simulink library "Renault_Library"  (Simulink 9.2)
settings: Solver=ode3  StartTime=0.0  StopTime=10.0  FixedStep=0.020  RelTol=1e-3  EnableMultiTasking=on
undefined symbols (not defined in the file or in its folder): ABC, AssystemProposal, b, chkval, CoeffA_C, CoeffB_C, CoeffMean_C, ConfTime_C, DiagDelay_C, DividePercent, FailureConfActive_C, FallingEdgeDuration_C, HighLevelThresholdVoltage_C, HighLevelVoltage_C, HighLevelVoltageTunable_C, InitValue_C, InputVoltRangeMax_C, InputVoltRangeMin_C, LowActiveContact_C, LowLevelThresholdVoltage_C, LowLevelVoltage_C, MaxOperatingVoltage_C, MinOperatingVoltage_C, mV2V_conversion, OUT1, OutOfRangeHigh_C, OutOfRangeLow_C, PinSupplySaturationMax, PinSupplySaturationMin, Res1Value_C, Res2Value_C, Res3Value_C, RisingEdgeDuration_C, RSA_CANSignalFailMonitoring, SampleTime_C, service14Table, service19Table, service22Table, service24Table, service28Table, service2ETable, service2FTable, service31Table, service87Table, SizeXStateDID2FTable, SizeXTable14, SizeXTable19, SizeXTable22, SizeXTable24, SizeXTable28, SizeXTable2E, SizeXTable2F, SizeXTable31, SizeXTable87, SizeYStateDID2FTable, SizeYTable14, SizeYTable19, SizeYTable22, SizeYTable24, SizeYTable28 ... (+22)
undefined symbols (not defined in the file): BatteryVoltage_ActivationThreshold_C, FrameStatus_AbsentValue_C, FrameStatus_ConfAbsentValue_C, MemThreshold_C, NAMEOFTHEMODEL_SAMPLE_TIME_CST, NbAbs1_C, NbConfAbs1_C, NbConfPres1_C, NbPres1_C, NOMDUSIGNAL_FaultConfTime_C, NOMDUSIGNAL_FaultRehabTime_C, NOMDUSIGNAL_switchvalue_C, NOMDUSIGNAL_unavailablevalue_C, PeriodFrame1_C, ResetTime_C, WakeUpTime_C
libraries: $bdroot x112, simulink x75
(part 30 of 32)

== stateflow chart Services ECU/Diag/RSA_DiagnosticManagement_v02.00/DIAGNOSTIC_ACTION_TOP/Diagnostic_Action ==
  state Action/
    state No_Message/
      entry: RES_DATA_NUMBER=0;
    state Response/
      entry :
      serviceAction(0);...
      DIAG_BUFFER_RESPONSE[0]=DIAG_BUFFER_REQUEST[0]+0x40;
      during :serviceAction(1);
    state Message_without_response/
      entry:
      RES_DATA_NUMBER=1;
    state Negative_Response/
      entry :
      DIAG_BUFFER_RESPONSE[0]=0x7F;...
      DIAG_BUFFER_RESPONSE[1]=DIAG_BUFFER_REQUEST[0];...
      DIAG_BUFFER_RESPONSE[2]=NEGATIVE_RES_CODE;...
      RES_DATA_NUMBER=3;
    (default) -> No_Message
    (junction 10) -> No_Message
    Negative_Response -> No_Message : [hasChanged(RES_DATA_NUMBER)]
    Response -> No_Message : [hasChanged(RES_DATA_NUMBER) ||...
        |    hasChanged(DIAG_BUFFER_REQUEST)]
    (junction 13) -> (junction 10)
    No_Message -> (junction 11) : [hasChanged(SEND_RES_MESSAGE)]
        | {
        |  DIAG_BUFFER_RESPONSE=0;...
        |
        | }
    (junction 11) -> Negative_Response [order 1] : [NEGATIVE_RES_CODE!=0]
    (junction 11) -> Response [order 3] : [NEGATIVE_RES_CODE==0 &&...
        |  SUPPRESS_POS_RES!=1]
    (junction 11) -> Message_without_response [order 2] : [NEGATIVE_RES_CODE==0 &&...
        |    SUPPRESS_POS_RES==1]
    Message_without_response -> (junction 12)
    (junction 12) -> (junction 13)
  (default) -> Action : {defineValues();}
  data: fillResponse function_input double; data_number local uint16; DIAG_BUFFER_REQUEST input uint8 size=4095; NEGATIVE_RES_CODE input uint8; SUPPRESS_POS_RES input double; DIAG_OUTPUT output Bus: busOutput size=1 =0; SEND_RES_MESSAGE input boolean; DIAG_BUFFER_RESPONSE output uint8 size=4095; RES_DATA_NUMBER output uint16; LIST_DID_SERVICE_22 input uint16 size=[2046 3]; DIAG_INPUT input Bus: busInput size=1; P2_server_max local uint16 =P2_server_max; P2server_max local uint16 =P2server_max
  function serviceAction(fillResponse):
    | function serviceAction(fillResponse)
    |     service=DIAG_BUFFER_REQUEST(1);
    |     %create a M-file for each service 
    |     switch service
    |         case (hex2dec('10'))
    |             [DIAG_OUTPUT,DIAG_BUFFER_RESPONSE,RES_DATA_NUMBER]=startDiagnosticSession(DIAG_OUTPUT,DIAG_BUFFER_RESPONSE,RES_DATA_NUMBER,DIAG_INPUT,DIAG_BUFFER_REQUEST,fillResponse);
    |             
    |         case (hex2dec('11'))
    |             [DIAG_OUTPUT,DIAG_BUFFER_RESPONSE,RES_DATA_NUMBER]= ecuReset(DIAG_OUTPUT,DIAG_BUFFER_RESPONSE,RES_DATA_NUMBER,DIAG_INPUT,DIAG_BUFFER_REQUEST,fillResponse);
    |             
    |         case (hex2dec('14'))
    |             [DIAG_OUTPUT,DIAG_BUFFER_RESPONSE,RES_DATA_NUMBER]= clearDTC(DIAG_OUTPUT,DIAG_BUFFER_RESPONSE,RES_DATA_NUMBER,DIAG_INPUT,DIAG_BUFFER_REQUEST,fillResponse);
    |             
    |         case (hex2dec('19'))
    |             subFunction = DIAG_BUFFER_REQUEST(2);
    |             [DIAG_BUFFER_RESPONSE,RES_DATA_NUMBER]=readDTCInformation(subFunction,DIAG_BUFFER_REQUEST,DIAG_BUFFER_RESPONSE,RES_DATA_NUMBER,DIAG_INPUT,fillResponse);
    |             
    |         case (hex2dec('22'))
    |             if(fillResponse==1)
    |                 data_number=uint16(0);
    |                 for current_did=1:2046
    |                     if( LIST_DID_SERVICE_22(current_did,1)~=0)
    |                         DIAG_BUFFER_RESPONSE=readDataByIdentifier(LIST_DID_SERVICE_22(current_did,1),(LIST_DID_SERVICE_22(current_did,2)-1)*8,DIAG_BUFFER_RESPONSE,DIAG_INPUT);
    |                         data_number =LIST_DID_SERVICE_22(current_did,2)+1+LIST_DID_SERVICE_22(current_did,3)+1;         
    |                     end                
    |                 end
    |                 RES_DATA_NUMBER=data_number;
    |             end
    |             
    |         case (hex2dec('23'))            
    |             [DIAG_BUFFER_RESPONSE,RES_DATA_NUMBER]= readMemoryByAddress(DIAG_BUFFER_RESPONSE,RES_DATA_NUMBER,DIAG_INPUT,DIAG_BUFFER_REQUEST,fillResponse);
    |         
    |         case (hex2dec('24'))            
    |             [DIAG_BUFFER_RESPONSE,RES_DATA_NUMBER]= readScalingDataByIdentifier(DIAG_BUFFER_RESPONSE,RES_DATA_NUMBER,DIAG_INPUT,DIAG_BUFFER_REQUEST,fillResponse);
    |               
    |         case (hex2dec('27'))
    |             [DIAG_OUTPUT,DIAG_BUFFER_RESPONSE,RES_DATA_NUMBER]= securityAccess(DIAG_OUTPUT,DIAG_BUFFER_RESPONSE,RES_DATA_NUMBER,DIAG_INPUT,DIAG_BUFFER_REQUEST,fillResponse);
    |         
    |         case (hex2dec('28'))
    |             [DIAG_OUTPUT,DIAG_BUFFER_RESPONSE,RES_DATA_NUMBER]= communicationControl(DIAG_OUTPUT,DIAG_BUFFER_RESPONSE,RES_DATA_NUMBER,DIAG_INPUT,DIAG_BUFFER_REQUEST,fillResponse);
    |         
    |         case (hex2dec('2E'))
    |             [DIAG_OUTPUT,DIAG_BUFFER_RESPONSE,RES_DATA_NUMBER]= writeDataByIdentifier(DIAG_OUTPUT,DIAG_BUFFER_RESPONSE,RES_DATA_NUMBER,DIAG_INPUT,DIAG_BUFFER_REQUEST,fillResponse);
    |         
    |         case (hex2dec('2F'))
    |             [DIAG_OUTPUT,DIAG_BUFFER_RESPONSE,RES_DATA_NUMBER]= inputOutputControl(DIAG_OUTPUT,DIAG_BUFFER_RESPONSE,RES_DATA_NUMBER,DIAG_INPUT,DIAG_BUFFER_REQUEST,fillResponse);
    |         
    |         case (hex2dec('31'))
    |             [DIAG_OUTPUT,DIAG_BUFFER_RESPONSE,RES_DATA_NUMBER]= startRoutine(DIAG_OUTPUT,DIAG_BUFFER_RESPONSE,RES_DATA_NUMBER,DIAG_INPUT,DIAG_BUFFER_REQUEST,fillResponse);
    |         
    |         case (hex2dec('3E'))
    |             RES_DATA_NUMBER=uint16(2);
    |             
    |         case (hex2dec('87'))
    |             [DIAG_OUTPUT,DIAG_BUFFER_RESPONSE,RES_DATA_NUMBER]= linkControl(DIAG_OUTPUT,DIAG_BUFFER_RESPONSE,RES_DATA_NUMBER,DIAG_INPUT,DIAG_BUFFER_REQUEST,fillResponse);
    | 
    |     end
    | end
  function defineValues:
    | function defineValues
    |      DIAG_OUTPUT=defineInitialValues(DIAG_OUTPUT);
    | end

== stateflow chart Services ECU/Diag/RSA_DiagnosticManagement_v02.00/DIAGNOSTIC_SANCTION_TOP/GeneralService/General_Server_Response ==
  (default) -> (junction 52) : [hasChanged(REQ_DATA_NUMBER) && REQ_DATA_NUMBER!=0]
      | {
      | NEGATIVE_RES_CODE=0x00;...
      | }
  (junction 50) -> (junction 51) [order 1] : [SERVICE_SUPPORTED_IN_ACTIVE_SESSION]
  (junction 53) -> (junction 50) [order 1] : [SERVICE_SUPPORTED]
  (junction 52) -> (junction 53) [order 1] : [ECU_AVAILABLE]
  (junction 50) -> (junction 56) [order 2] : {
      | NEGATIVE_RES_CODE= 0x7F ;...
      | SEND_RES_MESSAGE=!SEND_RES_MESSAGE;
      | }
  (junction 51) -> (junction 57) : {
      | NUMBER_SERVICE=DIAG_BUFFER_REQUEST[0];
      | REQUEST_RECEIVED=!REQUEST_RECEIVED;
      | }
  (junction 52) -> (junction 54) [order 2] : {
      | NEGATIVE_RES_CODE= 0x21 ;...
      | SEND_RES_MESSAGE=!SEND_RES_MESSAGE;
      | }
  (junction 53) -> (junction 55) [order 2] : {
      | NEGATIVE_RES_CODE= 0x11 ;...
      | SEND_RES_MESSAGE=!SEND_RES_MESSAGE;
      | }
  (junction 55) -> (junction 54)
  (junction 56) -> (junction 55)
  (junction 57) -> (junction 56)
  (junction 54) -> (junction 58)
  data: REQ_DATA_NUMBER input uint16; DIAG_BUFFER_REQUEST input uint8 size=4095; NEGATIVE_RES_CODE output uint8; SERVICE_SUPPORTED input boolean; SERVICE_SUPPORTED_IN_ACTIVE_SESSION input boolean; ECU_AVAILABLE input boolean; NUMBER_SERVICE output uint8; SEND_RES_MESSAGE output boolean; REQUEST_RECEIVED output boolean

== stateflow chart Services ECU/Diag/RSA_DiagnosticManagement_v02.00/DIAGNOSTIC_SANCTION_TOP/Service10/Check_Message_Service10 ==
  (default) -> (junction 78)
  (junction 78) -> (junction 79) [order 1] : [LENGTH_MESSAGE_CORRECT]
  (junction 79) -> (junction 80) [order 1] : [SUBFUNCTION_SUPPORTED]
  (junction 80) -> (junction 81) [order 1] : [CONDITION_CORRECT]
  (junction 78) -> (junction 82) [order 2] : {
      | NEGATIVE_RES_CODE= 0x13 ;
      | }
  (junction 79) -> (junction 83) [order 2] : {
      | NEGATIVE_RES_CODE= 0x12 ;
      | }
  (junction 80) -> (junction 84) [order 2] : {
      | NEGATIVE_RES_CODE= 0x22 ;
      | }
  (junction 81) -> (junction 85)
  (junction 83) -> (junction 82)
  (junction 84) -> (junction 83)
  (junction 85) -> (junction 84)
  (junction 82) -> (junction 86) : {
      |  SEND_RES_MESSAGE=!SEND_RES_MESSAGE;
      | }
  data: SUBFUNCTION_SUPPORTED input boolean; LENGTH_MESSAGE_CORRECT input boolean; CONDITION_CORRECT input boolean; NEGATIVE_RES_CODE output uint8; SEND_RES_MESSAGE output boolean

== stateflow chart Services ECU/Diag/RSA_DiagnosticManagement_v02.00/DIAGNOSTIC_SANCTION_TOP/Service11/Check_Message_Service11 ==
  (default) -> (junction 113)
  (junction 108) -> (junction 109) [order 1] : [SUBFUNCTION_SUPPORTED_IN_ACTIVE_SESSION]
  (junction 109) -> (junction 110) [order 1] : [SECURITY_ACCESS_ACCEPTED]
  (junction 110) -> (junction 111) [order 1] : [CONDITION_CORRECT]
  (junction 112) -> (junction 108) [order 1] : [SUBFUNCTION_SUPPORTED]
  (junction 113) -> (junction 112) [order 1] : [LENGTH_MESSAGE_CORRECT]
  (junction 108) -> (junction 116) [order 2] : {
      | NEGATIVE_RES_CODE= 0x7E ;
      | }
  (junction 109) -> (junction 117) [order 2] : {
      | NEGATIVE_RES_CODE= 0x33 ;
      | }
  (junction 110) -> (junction 118) [order 2] : {
      | NEGATIVE_RES_CODE= 0x22 ;
      | }
  (junction 111) -> (junction 119)
  (junction 112) -> (junction 115) [order 2] : {
      | NEGATIVE_RES_CODE= 0x12 ;
      | }
  (junction 113) -> (junction 114) [order 2] : {
      | NEGATIVE_RES_CODE= 0x13 ;
      | }
  (junction 115) -> (junction 114)
  (junction 116) -> (junction 115)
  (junction 117) -> (junction 116)
  (junction 118) -> (junction 117)
  (junction 119) -> (junction 118)
  (junction 114) -> (junction 120) : {
      |  SEND_RES_MESSAGE=!SEND_RES_MESSAGE;
      | }
  data: SUBFUNCTION_SUPPORTED input boolean; SUBFUNCTION_SUPPORTED_IN_ACTIVE_SESSION input boolean; LENGTH_MESSAGE_CORRECT input boolean; CONDITION_CORRECT input boolean; NEGATIVE_RES_CODE output uint8; SECURITY_ACCESS_ACCEPTED input boolean; SEND_RES_MESSAGE output boolean

== stateflow chart Services ECU/Diag/RSA_DiagnosticManagement_v02.00/DIAGNOSTIC_SANCTION_TOP/Service14/Check_Message_Service14 ==
  (default) -> (junction 149)
  (junction 146) -> (junction 147) [order 1] : [PARAMETER_VALID]
  (junction 147) -> (junction 148) [order 1] : [CONDITION_CORRECT]
  (junction 149) -> (junction 146) [order 1] : [LENGTH_MESSAGE_CORRECT]
  (junction 146) -> (junction 150) [order 2] : {
      | NEGATIVE_RES_CODE= 0x31 ;
      | }
  (junction 147) -> (junction 151) [order 2] : {
      | NEGATIVE_RES_CODE= 0x22 ;
      | }
  (junction 148) -> (junction 152)
  (junction 149) -> (junction 153) [order 2] : {
      | NEGATIVE_RES_CODE= 0x13 ;
      | }
  (junction 151) -> (junction 150)
  (junction 152) -> (junction 151)
  (junction 150) -> (junction 153)
  (junction 153) -> (junction 154) : {
      |  SEND_RES_MESSAGE=!SEND_RES_MESSAGE;
      | }
  data: LENGTH_MESSAGE_CORRECT input boolean; CONDITION_CORRECT input boolean; NEGATIVE_RES_CODE output uint8; PARAMETER_VALID input boolean; SEND_RES_MESSAGE output boolean

== stateflow chart Services ECU/Diag/RSA_DiagnosticManagement_v02.00/DIAGNOSTIC_SANCTION_TOP/Service14/Parameter_Valid_Service14/Parameter_service14 ==
  state Session [function]
    (default) -> (junction 170)
    (junction 170) -> (junction 171) [order 1] : [SESSION_ACTIVE==DEFAULT_SESSION]
        | {
        |  s=1;
        | }
    (junction 170) -> (junction 172) [order 2]
    (junction 171) -> (junction 173)
    (junction 172) -> (junction 173) [order 1] : [SESSION_ACTIVE==PROGRAMMING_SESSION]
        | {
        |  s=2;
        | }
    (junction 172) -> (junction 174) [order 2]
    (junction 173) -> (junction 175)
    (junction 174) -> (junction 175) [order 1] : [SESSION_ACTIVE==EXTENDED_SESSION]
        | {
        |  s=3;
        | }
    (junction 174) -> (junction 176) [order 2]
    (junction 175) -> (junction 177)
    (junction 176) -> (junction 177) [order 1] : [SESSION_ACTIVE==SAFETY_SYSTEM_SESSION]
        | {
        |  s=4;
        | }
    (junction 176) -> (junction 178) [order 2]
    (junction 177) -> (junction 179)
    (junction 179) -> (junction 178)
    (junction 178) -> (junction 180)
  (default) -> (junction 206) : {
      |  j=0;...
      |  PARAMETER_VALID= false;...
      | }
  (junction 204) -> (junction 205) [order 1] : [DIAG_BUFFER_REQUEST[1] == service14Table[j][0] &&...
      |    DIAG_BUFFER_REQUEST[2] == service14Table[j][1] &&...
      |    DIAG_BUFFER_REQUEST[3] == service14Table[j][2] &&...
      |         service14Table[j][s+2]==1]
  (junction 206) -> (junction 204) [order 1] : [j< SizeXTable14 ]
      | {
      |  Session();
      | }
  (junction 204) -> (junction 207) [order 2]
  (junction 205) -> (junction 208) : {
      |  PARAMETER_VALID= true;...
      | }
  (junction 207) -> (junction 206) : {
      |  j ++;
      | }
  (junction 206) -> (junction 209) [order 2]
  (junction 208) -> (junction 207)
  data: DIAG_BUFFER_REQUEST input uint8 size=4095; PARAMETER_VALID output boolean; j local uint32; service14Table parameter double size=[SizeXTable14 SizeYTable14]; SizeYTable14 parameter double; SizeXTable14 parameter double; SESSION_ACTIVE input Enum: TYP_SESSION_ACTIVE; s local double

== stateflow chart Services ECU/Diag/RSA_DiagnosticManagement_v02.00/DIAGNOSTIC_SANCTION_TOP/Service19/Check_Message_Service19 ==
  (default) -> (junction 229)
  (junction 226) -> (junction 227) [order 1] : [SUBFUNCTION_SUPPORTED_IN_ACTIVE_SESSION]
  (junction 227) -> (junction 228) [order 1] : [PARAMETER_VALID]
  (junction 230) -> (junction 226) [order 1] : [SUBFUNCTION_SUPPORTED]
  (junction 229) -> (junction 230) [order 1] : [LENGTH_MESSAGE_CORRECT]
  (junction 226) -> (junction 233) [order 2] : {
      | NEGATIVE_RES_CODE= 0x7E ;
      | }
  (junction 227) -> (junction 234) [order 2] : {
      | NEGATIVE_RES_CODE= 0x31 ;
      | }
  (junction 228) -> (junction 235)
  (junction 229) -> (junction 231) [order 2] : {
      | NEGATIVE_RES_CODE= 0x13 ;
      | }
  (junction 230) -> (junction 232) [order 2] : {
      | NEGATIVE_RES_CODE= 0x12 ;
      | }
  (junction 232) -> (junction 231)
  (junction 233) -> (junction 232)
  (junction 234) -> (junction 233)
  (junction 235) -> (junction 234)
  (junction 231) -> (junction 236) : {
      |  SEND_RES_MESSAGE=!SEND_RES_MESSAGE;
      | }
  data: LENGTH_MESSAGE_CORRECT input boolean; NEGATIVE_RES_CODE output uint8; SUBFUNCTION_SUPPORTED_IN_ACTIVE_SESSION input boolean; PARAMETER_VALID input boolean; SUBFUNCTION_SUPPORTED input boolean; SEND_RES_MESSAGE output boolean

== stateflow chart Services ECU/Diag/RSA_DiagnosticManagement_v02.00/DIAGNOSTIC_SANCTION_TOP/Service19/Parameter_Valid_Service19/Parameter_service19 ==
  (default) -> (junction 260) : {
      |  j=0;...
      |  PARAMETER_VALID= false;
      | }
  (junction 260) -> (junction 261) [order 1] : [DIAG_BUFFER_REQUEST[1]==0x01 ||  DIAG_BUFFER_REQUEST[1]==0x11 ||...
      |    DIAG_BUFFER_REQUEST[1]==0x12 ||  DIAG_BUFFER_REQUEST[1]==0x02 ||...
      |    DIAG_BUFFER_REQUEST[1]==0x0F ||  DIAG_BUFFER_REQUEST[1]==0x13 ||...
      |    DIAG_BUFFER_REQUEST[1]==0x03 ||  DIAG_BUFFER_REQUEST[1]==0x07 ||...
      |    DIAG_BUFFER_REQUEST[1]==0x08 ||  DIAG_BUFFER_REQUEST[1]==0x13 ||...
      |    DIAG_BUFFER_REQUEST[1]==0x0A ||  DIAG_BUFFER_REQUEST[1]==0x0B ||...
      |    DIAG_BUFFER_REQUEST[1]==0x0C ||  DIAG_BUFFER_REQUEST[1]==0x0D ||...
      |    DIAG_BUFFER_REQUEST[1]==0x0E || DIAG_BUFFER_REQUEST[1]==0x14 ||...
      |                                                              DIAG_BUFFER_REQUEST[1]==0x15]
  (junction 260) -> (junction 262) [order 2]
  (junction 261) -> (junction 263) : {
      |  PARAMETER_VALID= true;...
      | }
  (junction 263) -> (junction 262)
  (junction 262) -> (junction 267)
  (junction 264) -> (junction 265) [order 1] : [DIAG_BUFFER_REQUEST[2]== service19Table[j][0] &&...
      |         DIAG_BUFFER_REQUEST[3]== service19Table[j][1] &&...
      |        DIAG_BUFFER_REQUEST[4]== service19Table[j][2] ]
  (junction 265) -> (junction 266) [order 1] : [DIAG_BUFFER_REQUEST[1]==0x04 &&...
      |     DIAG_BUFFER_REQUEST[5]==service19Table[j][3]]
  (junction 268) -> (junction 264) [order 1] : [j< SizeXTable19 ]
  (junction 264) -> (junction 271) [order 2]
  (junction 265) -> (junction 269) [order 2]
  (junction 266) -> (junction 279) : {
      |  PARAMETER_VALID= true;
      | }
  (junction 267) -> (junction 268) [order 1] : [DIAG_BUFFER_REQUEST[1]==0x04 ||  DIAG_BUFFER_REQUEST[1]==0x09 ||...
      |    DIAG_BUFFER_REQUEST[1]==0x10 ||  DIAG_BUFFER_REQUEST[1]==0x06]
  (junction 271) -> (junction 268) : {
      |  j ++;
      | }
  (junction 267) -> (junction 274) [order 2]
  (junction 268) -> (junction 275) [order 2]
  (junction 269) -> (junction 270) [order 1] : [(DIAG_BUFFER_REQUEST[1]==0x06 ||...
      |    DIAG_BUFFER_REQUEST[1]==0x10) &&...
      |     DIAG_BUFFER_REQUEST[5]==0xFF]
  (junction 269) -> (junction 272) [order 2]
  (junction 270) -> (junction 278) : {
      |  PARAMETER_VALID= true;
      | }
  (junction 272) -> (junction 273) [order 1] : [DIAG_BUFFER_REQUEST[1]==0x09]
  (junction 276) -> (junction 271)
  (junction 275) -> (junction 274)
  (junction 272) -> (junction 276) [order 2]
  (junction 273) -> (junction 277) : {
      |  PARAMETER_VALID= true;
      | }
  (junction 274) -> (junction 280)
  (junction 277) -> (junction 276)
  (junction 278) -> (junction 277)
  (junction 279) -> (junction 278)
  data: DIAG_BUFFER_REQUEST input uint8 size=4095; PARAMETER_VALID output boolean; j local uint32; service19Table parameter double size=[SizeXTable19 SizeYTable19]; SizeXTable19 parameter double; SizeYTable19 parameter double

== stateflow chart Services ECU/Diag/RSA_DiagnosticManagement_v02.00/DIAGNOSTIC_SANCTION_TOP/Service22/Check_Message_Service22 ==
  (default) -> (junction 323)
  (junction 318) -> (junction 319) [order 1] : [PARAMETER_VALID]
  (junction 319) -> (junction 320) [order 1] : [SECURITY_ACCESS_ACCEPTED]
  (junction 320) -> (junction 321) [order 1] : [CONDITION_CORRECT]
  (junction 321) -> (junction 322) [order 1] : [LENGTH_RESPONSE_CORRECT]
  (junction 323) -> (junction 318) [order 1] : [LENGTH_MESSAGE_CORRECT]
  (junction 318) -> (junction 324) [order 2] : {
      | NEGATIVE_RES_CODE= 0x31 ;
      | }
  (junction 319) -> (junction 325) [order 2] : {
      | NEGATIVE_RES_CODE= 0x33 ;
      | }
  (junction 320) -> (junction 326) [order 2] : {
      | NEGATIVE_RES_CODE= 0x22 ;
      | }
  (junction 321) -> (junction 327) [order 2] : {
      | NEGATIVE_RES_CODE= 0x14 ;
      | }
  (junction 322) -> (junction 328)
  (junction 323) -> (junction 329) [order 2] : {
      | NEGATIVE_RES_CODE= 0x13 ;
      | }
  (junction 325) -> (junction 324)
  (junction 326) -> (junction 325)
  (junction 327) -> (junction 326)
  (junction 328) -> (junction 327)
  (junction 324) -> (junction 329)
  (junction 329) -> (junction 330) : {
      |  SEND_RES_MESSAGE=!SEND_RES_MESSAGE;
      | }
  data: LENGTH_MESSAGE_CORRECT input boolean; CONDITION_CORRECT input boolean; NEGATIVE_RES_CODE output uint8; LENGTH_RESPONSE_CORRECT input boolean; SECURITY_ACCESS_ACCEPTED input boolean; PARAMETER_VALID input boolean; SEND_RES_MESSAGE output boolean

== stateflow chart Services ECU/Diag/RSA_DiagnosticManagement_v02.00/DIAGNOSTIC_SANCTION_TOP/Service22/Parameter_Valid_Service22/Parameter_service22 ==
  state Session [function]
    (default) -> (junction 353)
    (junction 353) -> (junction 354) [order 1] : [SESSION_ACTIVE==DEFAULT_SESSION]
        | {
        |  s=1;
        | }
    (junction 353) -> (junction 355) [order 2]
    (junction 354) -> (junction 356)
    (junction 355) -> (junction 356) [order 1] : [SESSION_ACTIVE==PROGRAMMING_SESSION]
        | {
        |  s=2;
        | }
    (junction 355) -> (junction 357) [order 2]
    (junction 356) -> (junction 358)
    (junction 357) -> (junction 358) [order 1] : [SESSION_ACTIVE==EXTENDED_SESSION]
        | {
        |  s=3;
        | }
    (junction 357) -> (junction 359) [order 2]
    (junction 358) -> (junction 360)
    (junction 359) -> (junction 360) [order 1] : [SESSION_ACTIVE==SAFETY_SYSTEM_SESSION]
        | {
        |  s=4;
        | }
    (junction 359) -> (junction 361) [order 2]
    (junction 360) -> (junction 362)
    (junction 362) -> (junction 361)
    (junction 361) -> (junction 363)
  state Max_data_size_bit [function]
    (default) -> (junction 379)
    (junction 379) -> (junction 380) [order 1] : [DATA_SIZE_BIT < (service22Table[j][6]+service22Table[j][7]-1)]
    (junction 379) -> (junction 381) [order 2]
    (junction 380) -> (junction 382) : {
        |  DATA_SIZE_BIT=(service22Table[j][6]+service22Table[j][7]-1);
        | }
    (junction 382) -> (junction 381)
    (junction 381) -> (junction 383)
  (default) -> (junction 409) : {
      |  i=0;...
      |  j=0;...
      |  w=0;...
      |  SECURITY_ACCESS_ACCEPTED=true;...
      |  PARAMETER_VALID= false;...
      |  DATA_SIZE_BIT=0;...
      |  LIST_DID_SERVICE_22=0;...
      |  RESPONSE_SIZE=2;
      | }
  (junction 409) -> (junction 406) [order 1] : [i<((REQ_DATA_NUMBER-1))]
  (junction 406) -> (junction 414) : {
      |  DID=(DIAG_BUFFER_REQUEST[i+1]<<8) + DIAG_BUFFER_REQUEST[i+2];
      | }
  (junction 407) -> (junction 408) [order 1] : [DID == service22Table[j][0] &&...
      |  service22Table[j][s]==1]
  (junction 411) -> (junction 407) [order 1] : [j< SizeXTable22 ]
  (junction 407) -> (junction 415) [order 2]
  (junction 408) -> (junction 412) : {
      |  Max_data_size_bit();
      | }
  (junction 414) -> (junction 409) [order 2] : {
      |  i =i+2;...
      |  DATA_SIZE_BIT=0;...
      |  j=0;
      | }
  (junction 409) -> (junction 420) [order 2]
  (junction 410) -> (junction 411) [order 1] : [DID == service22Table[j][0] &&...
      |  service22Table[j][s]==1]
  (junction 412) -> (junction 413) [order 1] : [service22Table[j][5]==0]
  (junction 414) -> (junction 410) [order 1] : [j< SizeXTable22]
      | {
      |  Session();
      | }
  (junction 415) -> (junction 411) : {
      |  j ++;
      | }
  (junction 410) -> (junction 418) [order 2]
  (junction 411) -> (junction 419) [order 2] : {
      |  PARAMETER_VALID= true;...
      |  LIST_DID_SERVICE_22[w][0] = DID;...
      |  LIST_DID_SERVICE_22[w][1] = RESPONSE_SIZE-1;...
      |  LIST_DID_SERVICE_22[w][2] =(DATA_SIZE_BIT+7)/8-3;...
      |  w ++;...
      |  RESPONSE_SIZE=RESPONSE_SIZE+((DATA_SIZE_BIT+7)/8)-1 ;
      | }
  (junction 412) -> (junction 416) [order 2]
  (junction 413) -> (junction 417) : {
      |  SECURITY_ACCESS_ACCEPTED=false;
      | }
  (junction 418) -> (junction 414) : {
      |  j ++;
      | }
  (junction 416) -> (junction 415)
  (junction 417) -> (junction 416)
  (junction 419) -> (junction 418)
  data: DIAG_BUFFER_REQUEST input uint8 size=4095; REQ_DATA_NUMBER input uint8; PARAMETER_VALID output boolean; LIST_DID_SERVICE_22 output uint16 size=[2046 3]; DID local uint16; w local uint32; i local uint32; j local uint32; service22Table parameter double size=[SizeXTable22 SizeYTable22]; SizeYTable22 parameter double; SizeXTable22 parameter double; RESPONSE_SIZE output uint16; SECURITY_ACCESS_ACCEPTED output boolean; SESSION_ACTIVE input Enum: TYP_SESSION_ACTIVE; s local double; DATA_SIZE_BIT local double

== stateflow chart Services ECU/Diag/RSA_DiagnosticManagement_v02.00/DIAGNOSTIC_SANCTION_TOP/Service23/Check_Message_Service23 ==
  (default) -> (junction 450)
  (junction 450) -> (junction 451) [order 1] : [LENGTH_MESSAGE_CORRECT]
  (junction 451) -> (junction 452) [order 1] : [PARAMETER_VALID]
  (junction 452) -> (junction 453) [order 1] : [SECURITY_ACCESS_ACCEPTED]
  (junction 453) -> (junction 454) [order 1] : [CONDITION_CORRECT]
  (junction 450) -> (junction 459) [order 2] : {
      | NEGATIVE_RES_CODE= 0x13 ;
      | }
  (junction 451) -> (junction 455) [order 2] : {
      | NEGATIVE_RES_CODE= 0x31 ;
      | }
  (junction 452) -> (junction 456) [order 2] : {
      | NEGATIVE_RES_CODE= 0x33 ;
      | }
  (junction 453) -> (junction 457) [order 2] : {
      | NEGATIVE_RES_CODE= 0x22 ;
      | }
  (junction 454) -> (junction 458)
  (junction 456) -> (junction 455)
  (junction 457) -> (junction 456)
  (junction 458) -> (junction 457)
  (junction 455) -> (junction 459)
  (junction 459) -> (junction 460) : {
      |  SEND_RES_MESSAGE=!SEND_RES_MESSAGE;
      | }
  data: LENGTH_MESSAGE_CORRECT input boolean; CONDITION_CORRECT input boolean; NEGATIVE_RES_CODE output uint8; SECURITY_ACCESS_ACCEPTED input boolean; PARAMETER_VALID input boolean; SEND_RES_MESSAGE output boolean

== stateflow chart Services ECU/Commonly_Used_Blocks/RSA_ServECU_SoftwareFiltering/SWFilteringChart ==
  state StableState
  state ConfirmedState
  (default) -> StableState : /filtered_value=InitValue;
  (junction 485) -> StableState [order 2] : [raw_value==filtered_value]
  StableState -> ConfirmedState : [raw_value!=filtered_value]
  ConfirmedState -> StableState [order 1] : [after(Filter/SampleTime,tick)]
      | /filtered_value=raw_value;
  (junction 485) -> ConfirmedState [order 1] : [raw_value!=filtered_value]
  ConfirmedState -> (junction 485) [order 2] : [hasChanged(raw_value)]
  data: raw_value input double; Filter input double; SampleTime input double; filtered_value output double =4; InitValue input double =InitValue_C

== stateflow chart Services ECU/Diag/RSA_DiagnosticManagement_v02.00/DIAGNOSTIC_SANCTION_TOP/Service23/Parameter_Valid_Service23/Parameter_service23 ==
  state Session [function]
    (default) -> (junction 495)
    (junction 495) -> (junction 496) [order 1] : [SESSION_ACTIVE==DEFAULT_SESSION]
        | {
        |  s=1;
        | }
    (junction 495) -> (junction 497) [order 2]
    (junction 496) -> (junction 498)
    (junction 497) -> (junction 498) [order 1] : [SESSION_ACTIVE==PROGRAMMING_SESSION]
        | {
        |  s=2;
        | }
    (junction 497) -> (junction 499) [order 2]
    (junction 498) -> (junction 500)
    (junction 499) -> (junction 500) [order 1] : [SESSION_ACTIVE==EXTENDED_SESSION]
        | {
        |  s=3;
        | }
    (junction 499) -> (junction 501) [order 2]
    (junction 500) -> (junction 502)
    (junction 501) -> (junction 502) [order 1] : [SESSION_ACTIVE==SAFETY_SYSTEM_SESSION]
        | {
        |  s=4;
        | }
    (junction 501) -> (junction 503) [order 2]
    (junction 502) -> (junction 504)
    (junction 504) -> (junction 503)
    (junction 503) -> (junction 505)
  (default) -> (junction 530) : {
      |  i=1;...
      |  SECURITY_ACCESS_ACCEPTED=false;...
      |  PARAMETER_VALID= false;...
      |  memorySize=0;
      | }
  (junction 530) -> (junction 529) [order 1] : [i<=(DIAG_BUFFER_REQUEST[1]&0x0F)]
  (junction 529) -> (junction 531) : {
      |  memorySize+=DIAG_BUFFER_REQUEST[REQ_DATA_NUMBER-i]<<((i-1)*8);
      | }
  (junction 531) -> (junction 530) : {
      |  i ++;
      | }
  (junction 530) -> (junction 532) [order 2]
  (junction 532) -> (junction 533) [order 1] : [memorySize!=0 && ...
      |  memorySize<4095]
  (junction 532) -> (junction 534) [order 2]
  (junction 533) -> (junction 535) : {
      |  PARAMETER_VALID=true;
      | }
  (junction 535) -> (junction 534)
  (junction 534) -> (junction 536)
  data: DIAG_BUFFER_REQUEST input uint8 size=4095; REQ_DATA_NUMBER input uint8; PARAMETER_VALID output boolean; i local uint32; SECURITY_ACCESS_ACCEPTED output boolean; SESSION_ACTIVE input Enum: TYP_SESSION_ACTIVE; s local double; memorySize local double

== stateflow chart Services ECU/Diag/RSA_DiagnosticManagement/DIAGNOSTIC_ACTION_TOP/Diagnostic_Action ==
  state Action/
    state No_Message/
      entry: RES_DATA_NUMBER=0;
    state Response/
      entry :
      serviceAction(0);...
      DIAG_BUFFER_RESPONSE[0]=DIAG_BUFFER_REQUEST[0]+0x40;
      during :serviceAction(1);
    state Message_without_response/
      entry:
      RES_DATA_NUMBER=1;
    state Negative_Response/
      entry :
      DIAG_BUFFER_RESPONSE[0]=0x7F;...
      DIAG_BUFFER_RESPONSE[1]=DIAG_BUFFER_REQUEST[0];...
      DIAG_BUFFER_RESPONSE[2]=NEGATIVE_RES_CODE;...
      RES_DATA_NUMBER=3;
    (default) -> No_Message
    (junction 556) -> No_Message
    Negative_Response -> No_Message : [hasChanged(RES_DATA_NUMBER)]
    Response -> No_Message : [hasChanged(RES_DATA_NUMBER) ||...
        |    hasChanged(DIAG_BUFFER_REQUEST)]
    (junction 559) -> (junction 556)
    No_Message -> (junction 557) : [hasChanged(SEND_RES_MESSAGE)]
        | {
        |  DIAG_BUFFER_RESPONSE=0;...
        |
        | }
    (junction 557) -> Negative_Response [order 1] : [NEGATIVE_RES_CODE!=0]
    (junction 557) -> Response [order 3] : [NEGATIVE_RES_CODE==0 &&...
        |  SUPPRESS_POS_RES!=1]
    (junction 557) -> Message_without_response [order 2] : [NEGATIVE_RES_CODE==0 &&...
        |    SUPPRESS_POS_RES==1]
    Message_without_response -> (junction 558)
    (junction 558) -> (junction 559)
  (default) -> Action : {defineValues();}
  data: fillResponse function_input double; data_number local uint16; DIAG_BUFFER_REQUEST input uint8 size=4095; NEGATIVE_RES_CODE input uint8; SUPPRESS_POS_RES input double; DIAG_OUTPUT output Bus: busOutput size=1 =0; SEND_RES_MESSAGE input boolean; DIAG_BUFFER_RESPONSE output uint8 size=4095; RES_DATA_NUMBER output uint16; LIST_DID_SERVICE_22 input uint16 size=[2046 3]; DIAG_INPUT input Bus: busInput size=1; P2_server_max local uint16 =P2_server_max; P2server_max local uint16 =P2server_max
  function serviceAction(fillResponse):
    | function serviceAction(fillResponse)
    |     service=DIAG_BUFFER_REQUEST(1);
    |     %create a M-file for each service 
    |     switch service
    |         case (hex2dec('10'))
    |             [DIAG_OUTPUT,DIAG_BUFFER_RESPONSE,RES_DATA_NUMBER]=startDiagnosticSession(DIAG_OUTPUT,DIAG_BUFFER_RESPONSE,RES_DATA_NUMBER,DIAG_INPUT,DIAG_BUFFER_REQUEST,fillResponse);
    |             
    |         case (hex2dec('11'))
    |             [DIAG_OUTPUT,DIAG_BUFFER_RESPONSE,RES_DATA_NUMBER]= ecuReset(DIAG_OUTPUT,DIAG_BUFFER_RESPONSE,RES_DATA_NUMBER,DIAG_INPUT,DIAG_BUFFER_REQUEST,fillResponse);
    |             
    |         case (hex2dec('14'))
    |             [DIAG_OUTPUT,DIAG_BUFFER_RESPONSE,RES_DATA_NUMBER]= clearDTC(DIAG_OUTPUT,DIAG_BUFFER_RESPONSE,RES_DATA_NUMBER,DIAG_INPUT,DIAG_BUFFER_REQUEST,fillResponse);
    |             
    |         case (hex2dec('19'))
    |             subFunction = DIAG_BUFFER_REQUEST(2);
    |             [DIAG_BUFFER_RESPONSE,RES_DATA_NUMBER]=readDTCInformation(subFunction,DIAG_BUFFER_REQUEST,DIAG_BUFFER_RESPONSE,RES_DATA_NUMBER,DIAG_INPUT,fillResponse);
    |             
    |         case (hex2dec('22'))
    |             if(fillResponse==1)
    |                 data_number=uint16(0);
    |                 for current_did=1:2046
    |                     if( LIST_DID_SERVICE_22(current_did,1)~=0)
    |                         DIAG_BUFFER_RESPONSE=readDataByIdentifier(LIST_DID_SERVICE_22(current_did,1),(LIST_DID_SERVICE_22(current_did,2)-1)*8,DIAG_BUFFER_RESPONSE,DIAG_INPUT);
    |                         data_number =LIST_DID_SERVICE_22(current_did,2)+1+LIST_DID_SERVICE_22(current_did,3)+1;         
    |                     end                
    |                 end
    |                 RES_DATA_NUMBER=data_number;
    |             end
    |             
    |         case (hex2dec('23'))            
    |             [DIAG_BUFFER_RESPONSE,RES_DATA_NUMBER]= readMemoryByAddress(DIAG_BUFFER_RESPONSE,RES_DATA_NUMBER,DIAG_INPUT,DIAG_BUFFER_REQUEST,fillResponse);
    |         
    |         case (hex2dec('24'))            
    |             [DIAG_BUFFER_RESPONSE,RES_DATA_NUMBER]= readScalingDataByIdentifier(DIAG_BUFFER_RESPONSE,RES_DATA_NUMBER,DIAG_INPUT,DIAG_BUFFER_REQUEST,fillResponse);
    |               
    |         case (hex2dec('27'))
    |             [DIAG_OUTPUT,DIAG_BUFFER_RESPONSE,RES_DATA_NUMBER]= securityAccess(DIAG_OUTPUT,DIAG_BUFFER_RESPONSE,RES_DATA_NUMBER,DIAG_INPUT,DIAG_BUFFER_REQUEST,fillResponse);
    |         
    |         case (hex2dec('28'))
    |             [DIAG_OUTPUT,DIAG_BUFFER_RESPONSE,RES_DATA_NUMBER]= communicationControl(DIAG_OUTPUT,DIAG_BUFFER_RESPONSE,RES_DATA_NUMBER,DIAG_INPUT,DIAG_BUFFER_REQUEST,fillResponse);
    |         
    |         case (hex2dec('2E'))
    |             [DIAG_OUTPUT,DIAG_BUFFER_RESPONSE,RES_DATA_NUMBER]= writeDataByIdentifier(DIAG_OUTPUT,DIAG_BUFFER_RESPONSE,RES_DATA_NUMBER,DIAG_INPUT,DIAG_BUFFER_REQUEST,fillResponse);
    |         
    |         case (hex2dec('2F'))
    |             [DIAG_OUTPUT,DIAG_BUFFER_RESPONSE,RES_DATA_NUMBER]= inputOutputControl(DIAG_OUTPUT,DIAG_BUFFER_RESPONSE,RES_DATA_NUMBER,DIAG_INPUT,DIAG_BUFFER_REQUEST,fillResponse);
    |         
    |         case (hex2dec('31'))
    |             [DIAG_OUTPUT,DIAG_BUFFER_RESPONSE,RES_DATA_NUMBER]= startRoutine(DIAG_OUTPUT,DIAG_BUFFER_RESPONSE,RES_DATA_NUMBER,DIAG_INPUT,DIAG_BUFFER_REQUEST,fillResponse);
    |         
    |         case (hex2dec('3E'))
    |             RES_DATA_NUMBER=uint16(1);
    |             
    |         case (hex2dec('87'))
    |             [DIAG_OUTPUT,DIAG_BUFFER_RESPONSE,RES_DATA_NUMBER]= linkControl(DIAG_OUTPUT,DIAG_BUFFER_RESPONSE,RES_DATA_NUMBER,DIAG_INPUT,DIAG_BUFFER_REQUEST,fillResponse);
    | 
    |     end
    | end
  function defineValues:
    | function defineValues
    |      DIAG_OUTPUT=defineInitialValues(DIAG_OUTPUT);
    | end

== stateflow chart Services ECU/Diag/RSA_DiagnosticManagement/DIAGNOSTIC_SANCTION_TOP/GeneralService/General_Server_Response ==
  (default) -> (junction 598) : [hasChanged(REQ_DATA_NUMBER) && REQ_DATA_NUMBER!=0]
      | {
      | NEGATIVE_RES_CODE=0x00;...
      | }
  (junction 596) -> (junction 597) [order 1] : [SERVICE_SUPPORTED_IN_ACTIVE_SESSION]
  (junction 599) -> (junction 596) [order 1] : [SERVICE_SUPPORTED]
  (junction 598) -> (junction 599) [order 1] : [ECU_AVAILABLE]
  (junction 596) -> (junction 602) [order 2] : {
      | NEGATIVE_RES_CODE= 0x7F ;...
      | SEND_RES_MESSAGE=!SEND_RES_MESSAGE;
      | }
  (junction 597) -> (junction 603) : {
      | NUMBER_SERVICE=DIAG_BUFFER_REQUEST[0];
      | REQUEST_RECEIVED=!REQUEST_RECEIVED;
      | }
  (junction 598) -> (junction 600) [order 2] : {
      | NEGATIVE_RES_CODE= 0x21 ;...
      | SEND_RES_MESSAGE=!SEND_RES_MESSAGE;
      | }
  (junction 599) -> (junction 601) [order 2] : {
      | NEGATIVE_RES_CODE= 0x11 ;...
      | SEND_RES_MESSAGE=!SEND_RES_MESSAGE;
      | }
  (junction 601) -> (junction 600)
  (junction 602) -> (junction 601)
  (junction 603) -> (junction 602)
  (junction 600) -> (junction 604)
  data: REQ_DATA_NUMBER input uint16; DIAG_BUFFER_REQUEST input uint8 size=4095; NEGATIVE_RES_CODE output uint8; SERVICE_SUPPORTED input boolean; SERVICE_SUPPORTED_IN_ACTIVE_SESSION input boolean; ECU_AVAILABLE input boolean; NUMBER_SERVICE output uint8; SEND_RES_MESSAGE output boolean; REQUEST_RECEIVED output boolean

== stateflow chart Services ECU/Diag/RSA_DiagnosticManagement/DIAGNOSTIC_SANCTION_TOP/Service31/Check_Message_Service31 ==
  (default) -> (junction 634)
  (junction 635) -> (junction 628) [order 1] : [SUBFUNCTION_SUPPORTED]
  (junction 628) -> (junction 629) [order 1] : [SUBFUNCTION_SUPPORTED_IN_ACTIVE_SESSION]
  (junction 629) -> (junction 630) [order 1] : [PARAMETER_VALID]
  (junction 630) -> (junction 631) [order 1] : [SECURITY_ACCESS_ACCEPTED]
  (junction 631) -> (junction 632) [order 1] : [CONDITION_CORRECT]
  (junction 632) -> (junction 633) [order 1] : [REQ_SEQUENCE_OK]
  (junction 634) -> (junction 635) [order 1] : [LENGTH_MESSAGE_CORRECT]
  (junction 628) -> (junction 641) [order 2] : {
      | NEGATIVE_RES_CODE= 0x7E ;
      | }
  (junction 629) -> (junction 642) [order 2] : {
      | NEGATIVE_RES_CODE= 0x31
      | }
  (junction 630) -> (junction 643) [order 2] : {
      | NEGATIVE_RES_CODE= 0x33 ;
      | }
  (junction 631) -> (junction 637) [order 2] : {
      | NEGATIVE_RES_CODE= 0x22 ;
      | }
  (junction 632) -> (junction 638) [order 2] : {
      | NEGATIVE_RES_CODE= 0x24 ;
      | }
  (junction 633) -> (junction 639)
  (junction 634) -> (junction 636) [order 2] : {
      | NEGATIVE_RES_CODE= 0x13 ;
      | }
  (junction 635) -> (junction 640) [order 2] : {
      | NEGATIVE_RES_CODE= 0x12 ;
      | }
  (junction 640) -> (junction 636)
  (junction 638) -> (junction 637)
  (junction 639) -> (junction 638)
  (junction 637) -> (junction 643)
  (junction 641) -> (junction 640)
  (junction 642) -> (junction 641)
  (junction 643) -> (junction 642)
  (junction 636) -> (junction 644) : {
      |  SEND_RES_MESSAGE=!SEND_RES_MESSAGE;
      | }
  data: LENGTH_MESSAGE_CORRECT input boolean; REQ_SEQUENCE_OK input boolean; NEGATIVE_RES_CODE output uint8; CONDITION_CORRECT input boolean; SECURITY_ACCESS_ACCEPTED input boolean; PARAMETER_VALID input double; SUBFUNCTION_SUPPORTED_IN_ACTIVE_SESSION input boolean; SUBFUNCTION_SUPPORTED input boolean; SEND_RES_MESSAGE output boolean

== stateflow chart Services ECU/Diag/RSA_DiagnosticManagement/DIAGNOSTIC_SANCTION_TOP/Service3D/Parameter_Valid_Service3D/Parameter_service3D ==
  state Session [function]
    (default) -> (junction 672)
    (junction 672) -> (junction 673) [order 1] : [SESSION_ACTIVE==DEFAULT_SESSION]
        | {
        |  s=1;
        | }
    (junction 672) -> (junction 674) [order 2]
    (junction 673) -> (junction 675)
    (junction 674) -> (junction 675) [order 1] : [SESSION_ACTIVE==PROGRAMMING_SESSION]
        | {
        |  s=2;
        | }
    (junction 674) -> (junction 676) [order 2]
    (junction 675) -> (junction 677)
    (junction 676) -> (junction 677) [order 1] : [SESSION_ACTIVE==EXTENDED_SESSION]
        | {
        |  s=3;
        | }
    (junction 676) -> (junction 678) [order 2]
    (junction 677) -> (junction 679)
    (junction 678) -> (junction 679) [order 1] : [SESSION_ACTIVE==SAFETY_SYSTEM_SESSION]
        | {
        |  s=4;
        | }
    (junction 678) -> (junction 680) [order 2]
    (junction 679) -> (junction 681)
    (junction 681) -> (junction 680)
    (junction 680) -> (junction 682)
  (default) -> (junction 707) : {
      |  i=1;...
      |  SECURITY_ACCESS_ACCEPTED=false;...
      |  PARAMETER_VALID= false;...
      |  memorySize=0;
      | }
  (junction 707) -> (junction 706) [order 1] : [i<=(DIAG_BUFFER_REQUEST[1]&0x0F)]
  (junction 706) -> (junction 708) : {
      |  memorySize+=DIAG_BUFFER_REQUEST[REQ_DATA_NUMBER-i]<<((i-1)*8);
      | }
  (junction 708) -> (junction 707) : {
      |  i ++;
      | }
  (junction 707) -> (junction 709) [order 2]
  (junction 709) -> (junction 710) [order 1] : [memorySize!=0 && ...
      |  memorySize<4095]
  (junction 709) -> (junction 711) [order 2]
  (junction 710) -> (junction 712) : {
      |  PARAMETER_VALID=true;
      | }
  (junction 712) -> (junction 711)
  (junction 711) -> (junction 713)
  data: DIAG_BUFFER_REQUEST input uint8 size=4095; REQ_DATA_NUMBER input uint8; PARAMETER_VALID output boolean; i local uint32; SECURITY_ACCESS_ACCEPTED output boolean; SESSION_ACTIVE input Enum: TYP_SESSION_ACTIVE; s local double; memorySize local double

== stateflow chart Services ECU/Diag/RSA_DiagnosticManagement/DIAGNOSTIC_SEGMENTATION_TOP/Diagnostic_Segmentation ==
  state DIAG_SEGMENTATION
    state IDLE_STATE/
      entry :
      SEG_STATE = TYP_SEG_STATE.IDLE_STATE;...
      REQ_NUMBER=0;...
      RES_NUMBER=0;...
      REQ_DATA_NUMBER=0;...
      DIAG_BUFFER_REQUEST=0;
    state PROCESSING/
      entry :
      REQ_DATA_NUMBER=FRAME_LENGHT;...
      SEG_STATE = TYP_SEG_STATE.PROCESSING_IN_PROGRESS;
    state REQUEST_IN_PROGRESS/
      entry :
      SEG_STATE = TYP_SEG_STATE.RECEIVE_IN_PROGRESS;
      state WAITING_CONSECUTIVE_FRAME/
        entry :
        RES_FRAME_TYPE=3;...
        UpdateDiagResponse();...
        REQ_NUMBER=REQ_NUMBER+1;...
        REQ_BS=0;
      state ANALYSE_CONSECUTIVE_FRAME/
        entry :
        REQ_BS=REQ_BS+1;
      (default) -> WAITING_CONSECUTIVE_FRAME
      ANALYSE_CONSECUTIVE_FRAME -> WAITING_CONSECUTIVE_FRAME [order 2] : [(REQ_FRAME_NUMBER!=REQ_NUMBER) && ...
          |  REQ_FRAME_SN==(REQ_NUMBER & 0x000F) &&...
          |  RES_FRAME_BS!=0 && ...
          |  RES_FRAME_BS==REQ_BS]
      WAITING_CONSECUTIVE_FRAME -> (junction 760) [order 1] : [hasChanged(DTOOL_TO_ECU_FRAME_RECEIVED)]
          | /UpdateDiagRequest();
      (junction 760) -> REQUEST_IN_PROGRESS [order 3] : [REQ_FRAME_TYPE==1]
      (junction 760) -> ANALYSE_CONSECUTIVE_FRAME [order 2] : [REQ_FRAME_TYPE==2]
      (junction 761) -> ANALYSE_CONSECUTIVE_FRAME [order 2] : [REQ_FRAME_TYPE==2 &&...
          | (RES_FRAME_BS==0 || ...
          |  REQ_BS<RES_FRAME_BS)]
      ANALYSE_CONSECUTIVE_FRAME -> (junction 761) [order 1] : [hasChanged(DTOOL_TO_ECU_FRAME_RECEIVED)]
          | /UpdateDiagRequest();
      (junction 761) -> REQUEST_IN_PROGRESS [order 3] : [REQ_FRAME_TYPE==1]
    state RESPONSE_IN_PROGRESS/
      entry:
      SEG_STATE = TYP_SEG_STATE.TRANSMIT_IN_PROGRESS;
      state ANALYSE_FLOW_CONTROL/
        entry :
        RES_BS=0;
      state WAITING_FLOW_CONTROL/
        entry :
        RES_NUMBER=RES_NUMBER+1;...
        RES_BS=RES_BS+1;
      (junction 770) -> ANALYSE_FLOW_CONTROL : [REQ_FRAME_TYPE==3]
      ANALYSE_FLOW_CONTROL -> WAITING_FLOW_CONTROL [order 1] : after(REQ_FRAME_STMIN,tick)...
          | [REQ_FRAME_FS==0]
          | {RES_FRAME_TYPE=2;
          | UpdateDiagResponse();
          | }
      WAITING_FLOW_CONTROL -> (junction 770) [order 1] : [hasChanged(DTOOL_TO_ECU_FRAME_RECEIVED)]
      WAITING_FLOW_CONTROL -> WAITING_FLOW_CONTROL [order 3] : after(REQ_FRAME_STMIN,tick)
          | [RES_NUMBER<(RES_FRAME_NUMBER+1) && ...
          | (REQ_FRAME_BS==0 || ...
          |  RES_BS<REQ_FRAME_BS)]
          | {RES_FRAME_TYPE=2;...
          | UpdateDiagResponse();}
    (default) -> IDLE_STATE
    WAITING_FLOW_CONTROL -> IDLE_STATE [order 2] : [RES_NUMBER==(RES_FRAME_NUMBER+1)]
    ANALYSE_CONSECUTIVE_FRAME -> IDLE_STATE [order 4] : [REQ_FRAME_SN!=(REQ_NUMBER & 0x000F)]
    PROCESSING -> IDLE_STATE [order 2] : after(TIME_OUT_TM_CST,tick)
    (junction 739) -> IDLE_STATE [order 1] : [RES_DATA_NUMBER<=7 &&...
        |  DIAG_BUFFER_RESPONSE[0]!=0]
        | {RES_FRAME_TYPE=0;...
        | UpdateDiagResponse();
        | }
    (junction 739) -> IDLE_STATE [order 3] : [RES_DATA_NUMBER==1&&...
        |  DIAG_BUFFER_RESPONSE[0]==0]
        | {ECU_TO_DTOOL_BYTE_0=0;...
        |  ECU_TO_DTOOL_BYTE_1=0;...
        |  ECU_TO_DTOOL_BYTE_2=0;...
        |  ECU_TO_DTOOL_BYTE_3=0;...
        |  ECU_TO_DTOOL_BYTE_4=0;...
        |  ECU_TO_DTOOL_BYTE_5=0;...
        |  ECU_TO_DTOOL_BYTE_6=0;...
        |  ECU_TO_DTOOL_BYTE_7=0;
        | }
    ANALYSE_FLOW_CONTROL -> IDLE_STATE [order 2] : [REQ_FRAME_FS!=0]
    IDLE_STATE -> (junction 737) : [hasChanged(DTOOL_TO_ECU_FRAME_RECEIVED)]
        | /UpdateDiagRequest();
    (junction 737) -> REQUEST_IN_PROGRESS [order 2] : [REQ_FRAME_TYPE==1]
    (junction 737) -> PROCESSING [order 1] : [REQ_FRAME_TYPE==0]
    ANALYSE_FLOW_CONTROL -> DIAG_SEGMENTATION [order 3] : after(TIME_OUT_TM_CST,tick)
    WAITING_CONSECUTIVE_FRAME -> DIAG_SEGMENTATION [order 2] : after(TIME_OUT_TM_CST,tick)
    (junction 760) -> PROCESSING [order 1] : [REQ_FRAME_TYPE==0]
    PROCESSING -> (junction 738) [order 1] : [hasChanged(RES_DATA_NUMBER)]
    (junction 738) -> (junction 739) : [RES_DATA_NUMBER!=0]
    (junction 739) -> WAITING_FLOW_CONTROL [order 2] : [RES_DATA_NUMBER>7]
        | {RES_FRAME_NUMBER=(RES_DATA_NUMBER/7);...
        | RES_FRAME_TYPE=1;...
        | UpdateDiagResponse();
        | }
    ANALYSE_CONSECUTIVE_FRAME -> PROCESSING [order 3] : [REQ_FRAME_SN==(REQ_NUMBER & 0x0F)  && ...
        | REQ_FRAME_NUMBER==REQ_NUMBER]
    ANALYSE_CONSECUTIVE_FRAME -> DIAG_SEGMENTATION [order 5] : after(TIME_OUT_TM_CST,tick)
    WAITING_FLOW_CONTROL -> DIAG_SEGMENTATION [order 4] : after(TIME_OUT_TM_CST,tick)
    (junction 761) -> PROCESSING [order 1] : [REQ_FRAME_TYPE==0]
  state UpdateDiagResponse [function]
    (default) -> (junction 775)
    (junction 775) -> (junction 776) [order 1] : [RES_FRAME_TYPE==0]
        | {ECU_TO_DTOOL_BYTE_0=(RES_DATA_NUMBER & 0x000F);...
        |  ECU_TO_DTOOL_BYTE_1=DIAG_BUFFER_RESPONSE[0];...
        |  ECU_TO_DTOOL_BYTE_2=DIAG_BUFFER_RESPONSE[1];...
        |  ECU_TO_DTOOL_BYTE_3=DIAG_BUFFER_RESPONSE[2];...
        |  ECU_TO_DTOOL_BYTE_4=DIAG_BUFFER_RESPONSE[3];...
        |  ECU_TO_DTOOL_BYTE_5=DIAG_BUFFER_RESPONSE[4];...
        |  ECU_TO_DTOOL_BYTE_6=DIAG_BUFFER_RESPONSE[5];...
        |  ECU_TO_DTOOL_BYTE_7=DIAG_BUFFER_RESPONSE[6];
        | }
    (junction 775) -> (junction 777) [order 2]
    (junction 776) -> (junction 778)
    (junction 777) -> (junction 778) [order 1] : [RES_FRAME_TYPE==1]
        | {ECU_TO_DTOOL_BYTE_0=0x10+((RES_DATA_NUMBER & 0x0F00)>>8);...
        |  ECU_TO_DTOOL_BYTE_1=(RES_DATA_NUMBER & 0x00FF);...
        |  ECU_TO_DTOOL_BYTE_2=DIAG_BUFFER_RESPONSE[0];...
        |  ECU_TO_DTOOL_BYTE_3=DIAG_BUFFER_RESPONSE[1];...
        |  ECU_TO_DTOOL_BYTE_4=DIAG_BUFFER_RESPONSE[2];...
        |  ECU_TO_DTOOL_BYTE_5=DIAG_BUFFER_RESPONSE[3];...
        |  ECU_TO_DTOOL_BYTE_6=DIAG_BUFFER_RESPONSE[4];...
        |  ECU_TO_DTOOL_BYTE_7=DIAG_BUFFER_RESPONSE[5];
        | }
    (junction 777) -> (junction 779) [order 2]
    (junction 778) -> (junction 780)
    (junction 779) -> (junction 780) [order 1] : [RES_FRAME_TYPE==2]
        | {RES_FRAME_SN=RES_NUMBER;...
        |  ECU_TO_DTOOL_BYTE_0=0x20+(RES_FRAME_SN & 0x000F);...
        |  ECU_TO_DTOOL_BYTE_1=DIAG_BUFFER_RESPONSE[RES_FRAME_SN*7-1];...
        |  ECU_TO_DTOOL_BYTE_2=DIAG_BUFFER_RESPONSE[RES_FRAME_SN*7];...
        |  ECU_TO_DTOOL_BYTE_3=DIAG_BUFFER_RESPONSE[RES_FRAME_SN*7+1];...
        |  ECU_TO_DTOOL_BYTE_4=DIAG_BUFFER_RESPONSE[RES_FRAME_SN*7+2];...
        |  ECU_TO_DTOOL_BYTE_5=DIAG_BUFFER_RESPONSE[RES_FRAME_SN*7+3];...
        |  ECU_TO_DTOOL_BYTE_6=DIAG_BUFFER_RESPONSE[RES_FRAME_SN*7+4];...
        |  ECU_TO_DTOOL_BYTE_7=DIAG_BUFFER_RESPONSE[RES_FRAME_SN*7+5];
        | }
    (junction 779) -> (junction 781) [order 2]
    (junction 780) -> (junction 782)
    (junction 781) -> (junction 782) [order 1] : [RES_FRAME_TYPE==3]
        | {ECU_TO_DTOOL_BYTE_0=0x30+RES_FRAME_FS;...
        |  ECU_TO_DTOOL_BYTE_1=RES_FRAME_BS;...
        |  ECU_TO_DTOOL_BYTE_2=RES_FRAME_STMIN;...
        |  ECU_TO_DTOOL_BYTE_3=0x00;...
        |  ECU_TO_DTOOL_BYTE_4=0x00;...
        |  ECU_TO_DTOOL_BYTE_5=0x00;...
        |  ECU_TO_DTOOL_BYTE_6=0x00;...
        |  ECU_TO_DTOOL_BYTE_7=0x00;
        | }
    (junction 782) -> (junction 783) : {ECU_TO_DTOOL_SEND=ECU_TO_DTOOL_SEND+1;}
    (junction 781) -> (junction 783) [order 2]
  state UpdateDiagRequest [function]
    (default) -> (junction 797)
    (junction 797) -> (junction 798) [order 1] : [REQ_FRAME_TYPE==0]
        | {DIAG_BUFFER_REQUEST[0]=DTOOL_TO_ECU_BYTE_1;...
        |  DIAG_BUFFER_REQUEST[1]=DTOOL_TO_ECU_BYTE_2;...
        |  DIAG_BUFFER_REQUEST[2]=DTOOL_TO_ECU_BYTE_3;...
        |  DIAG_BUFFER_REQUEST[3]=DTOOL_TO_ECU_BYTE_4;...
        |  DIAG_BUFFER_REQUEST[4]=DTOOL_TO_ECU_BYTE_5;...
        |  DIAG_BUFFER_REQUEST[5]=DTOOL_TO_ECU_BYTE_6;...
        |  DIAG_BUFFER_REQUEST[6]=DTOOL_TO_ECU_BYTE_7;...
        |  FRAME_LENGHT=DTOOL_TO_ECU_BYTE_0 & 0x0F;
        |  }
    (junction 797) -> (junction 799) [order 2]
    (junction 798) -> (junction 800)
    (junction 799) -> (junction 800) [order 1] : [REQ_FRAME_TYPE==1]
        | {DIAG_BUFFER_REQUEST[0]=DTOOL_TO_ECU_BYTE_2;...
        |  DIAG_BUFFER_REQUEST[1]=DTOOL_TO_ECU_BYTE_3;...
        |  DIAG_BUFFER_REQUEST[2]=DTOOL_TO_ECU_BYTE_4;...
        |  DIAG_BUFFER_REQUEST[3]=DTOOL_TO_ECU_BYTE_5;...
        |  DIAG_BUFFER_REQUEST[4]=DTOOL_TO_ECU_BYTE_6;...
        |  DIAG_BUFFER_REQUEST[5]=DTOOL_TO_ECU_BYTE_7;...
        |  FRAME_LENGHT=((DTOOL_TO_ECU_BYTE_0 & 0x0F)<<8)+DTOOL_TO_ECU_BYTE_1;...
        |  REQ_FRAME_NUMBER=FRAME_LENGHT/7;
        |  }
    (junction 799) -> (junction 801) [order 2]
    (junction 800) -> (junction 802)
    (junction 801) -> (junction 802) [order 1] : [REQ_FRAME_TYPE==2]
        | {DIAG_BUFFER_REQUEST[REQ_NUMBER*7-1]=DTOOL_TO_ECU_BYTE_1;...
        |  DIAG_BUFFER_REQUEST[REQ_NUMBER*7]=DTOOL_TO_ECU_BYTE_2;...
        |  DIAG_BUFFER_REQUEST[REQ_NUMBER*7+1]=DTOOL_TO_ECU_BYTE_3;...
        |  DIAG_BUFFER_REQUEST[REQ_NUMBER*7+2]=DTOOL_TO_ECU_BYTE_4;...
        |  DIAG_BUFFER_REQUEST[REQ_NUMBER*7+3]=DTOOL_TO_ECU_BYTE_5;...
        |  DIAG_BUFFER_REQUEST[REQ_NUMBER*7+4]=DTOOL_TO_ECU_BYTE_6;......
        |  DIAG_BUFFER_REQUEST[REQ_NUMBER*7+5]=DTOOL_TO_ECU_BYTE_7;...
        |  REQ_FRAME_SN=DTOOL_TO_ECU_BYTE_0 & 0x0F;
        |  }
    (junction 801) -> (junction 803) [order 2]
    (junction 802) -> (junction 804)
    (junction 803) -> (junction 804) [order 1] : [REQ_FRAME_TYPE==3]
        | {REQ_FRAME_FS=DTOOL_TO_ECU_BYTE_0 & 0x0F;...
        |  REQ_FRAME_BS=DTOOL_TO_ECU_BYTE_1;...
        |  REQ_FRAME_STMIN=DTOOL_TO_ECU_BYTE_2;
        | }
    (junction 804) -> (junction 805)
    (junction 803) -> (junction 805) [order 2]
  data: DIAG_BUFFER_REQUEST output uint8 size=4095; REQ_DATA_NUMBER output uint16; ECU_TO_DTOOL_SEND output uint8; RES_FRAME_TYPE local uint8; DTOOL_TO_ECU_FRAME_RECEIVED input double; REQ_FRAME_TYPE input uint8; DTOOL_TO_ECU_BYTE_0 input uint8; TIME_OUT_TM_CST constant uint16 =90; REQ_BS local uint16 =0; REQ_NUMBER local uint16; RES_NUMBER local uint16; REQ_FRAME_NUMBER local uint8; REQ_FRAME_SN local uint8; REQ_FRAME_FS local uint8; REQ_FRAME_BS local uint8; REQ_FRAME_STMIN local uint8; SEG_STATE output Enum: TYP_SEG_STATE; RES_FRAME_BS constant uint8 =1; RES_FRAME_NUMBER local uint16; RES_BS local uint8; RES_FRAME_SN local uint16; DTOOL_TO_ECU_BYTE_1 input double; DTOOL_TO_ECU_BYTE_2 input double; DTOOL_TO_ECU_BYTE_3 input double; DTOOL_TO_ECU_BYTE_4 input double; DTOOL_TO_ECU_BYTE_5 input double; DTOOL_TO_ECU_BYTE_6 input double; DTOOL_TO_ECU_BYTE_7 input double; ECU_TO_DTOOL_BYTE_0 output uint8; ECU_TO_DTOOL_BYTE_1 output uint8; ECU_TO_DTOOL_BYTE_2 output uint8; ECU_TO_DTOOL_BYTE_3 output uint8; ECU_TO_DTOOL_BYTE_4 output uint8; ECU_TO_DTOOL_BYTE_5 output uint8; ECU_TO_DTOOL_BYTE_6 output uint8; ECU_TO_DTOOL_BYTE_7 output uint8; DIAG_BUFFER_RESPONSE input uint8 size=4095; RES_DATA_NUMBER input uint16; RES_FRAME_FS constant uint8 =0; RES_FRAME_STMIN constant uint8 =0; FRAME_LENGHT local uint16

== stateflow chart Services ECU/Mux/RSA_MuxMonitoring/CanFramePresence ==
  state ABSENT
    entry :
    FRAME_PRESENT = false;
    state CONFIRMATION_RUNNING
      entry : FRAME_STATUS = 2;
    state CONFIRMED_ABSENT
      entry:
      FRAME_STATUS = 3;
    (default) -> CONFIRMATION_RUNNING
    CONFIRMATION_RUNNING -> CONFIRMED_ABSENT : [after(CONFIRMED_ABSENT_PERIOD,tick)]
  state PRESENT
    entry :
    FRAME_STATUS = 1;
    FRAME_PRESENT = true;
  (default) -> ABSENT
  PRESENT -> ABSENT [order 2] : [after(3*FRAME_PERIOD,tick)]
  ABSENT -> PRESENT : [hasChanged(FRAME_RECEIVED)]
  PRESENT -> PRESENT [order 1] : [hasChanged(FRAME_RECEIVED)]
  data: FRAME_RECEIVED input uint32; FRAME_PERIOD parameter uint32; FRAME_STATUS output uint32; FRAME_PRESENT output boolean; CONFIRMED_ABSENT_PERIOD parameter uint32

== stateflow chart Services ECU/Mux/RSA_MuxSendFrame/RSA_Send_Frame_FCT ==
  state FRAME_MONITORING
    entry :
    Update_Counter();
  state Update_Counter [function]
    (default) -> (junction 881)
    (junction 881) -> (junction 882) [order 1] : [CNT_FRAME_UPDATED == MAX_PARAMETER]
    (junction 881) -> (junction 883) [order 2] : {CNT_FRAME_UPDATED = ...
        | CNT_FRAME_UPDATED + 1 ;}
    (junction 882) -> (junction 884) : {CNT_FRAME_UPDATED =0;}
    (junction 884) -> (junction 883)
    (junction 883) -> (junction 885)
  (default) -> FRAME_MONITORING : {FRAME_PRESENT = true;}
  FRAME_MONITORING -> FRAME_MONITORING [order 2] : [after(FRAME_PERIOD,tick)]
  FRAME_MONITORING -> FRAME_MONITORING [order 1] : [hasChangedTo(EVENT_DATA,true)]
  data: EVENT_DATA input boolean; FRAME_PERIOD parameter uint32; CNT_FRAME_UPDATED output uint32; FRAME_PRESENT output boolean =0; MAX_PARAMETER parameter uint32
